simulink library "R9000_FirmwareLib"  (R2024b)
settings: Solver=VariableStepAuto  StartTime=0.0  StopTime=10.0  RelTol=1e-3
libraries: mbd_s32k3_io_library x82, mbd_s32k3_isr_library x15, mbd_s32k3_communication_library x10, mbd_s32k3_utility_library x1
(part 7 of 7)

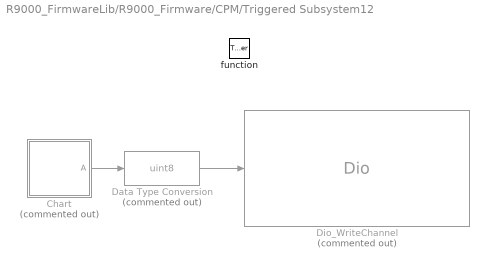
== system R9000_FirmwareLib/R9000_Firmware/CPM/Triggered Subsystem12 ==
  function : TriggerPort  TriggerType=function-call  VariantControl=Variant
  Chart : Stateflow chart  Commented=on  TreatAsAtomicUnit=on
  Data Type Conversion : DataTypeConversion  Commented=on  OutDataTypeStr=uint8  SaturateOnIntegerOverflow=off  RndMeth=Floor
  Dio_WriteChannel : mbd_s32k3_io_library/Dio  Commented=on  api_func=Dio_WriteChannel  channel=DI_DescentModeSwitch1  channel_group=No channel groups configured  port=PTA_H  gpioSim=on  text=<binary blob, 4879 chars, not shown>
      settings: SourceProductName=IO Blocks
  wires:
  Data Type Conversion/1 -> Dio_WriteChannel/1
  Chart/1(" A") -> Data Type Conversion/1

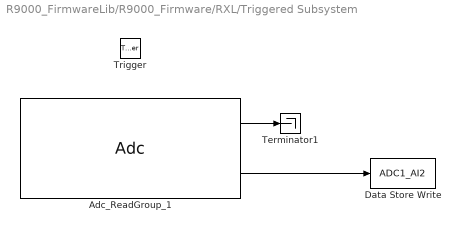
== system R9000_FirmwareLib/R9000_Firmware/RXL/Triggered Subsystem ==
  Trigger : TriggerPort  TriggerType=function-call  VariantControl=Variant
  Adc_ReadGroup_1 : mbd_s32k3_io_library/Adc  api_func=Adc_ReadGroup  hw_unit=AdcHwUnit_0  group=Adc0Group_0  channel=ADC_1_AI2  threshold_control=No ADC threshold control configured  power_state=ADC_FULL_POWER  ctu_unit=BctuHwUnit_0  ctu_trigger=AdcHwTrigger_0  ctu_fifo_idx=BctuResultFifos_0  text=<binary blob, 63510 chars, not shown>
      settings: SourceProductName=IO Blocks
  Data Store Write : DataStoreWrite  DataStoreName=ADC1_AI2  IndexOptions=Index vector (dialog)  Indices=1  OutputSizes=1
  Terminator1 : Terminator
  wires:
  Adc_ReadGroup_1/2 -> Data Store Write/1
  Adc_ReadGroup_1/1 -> Terminator1/1

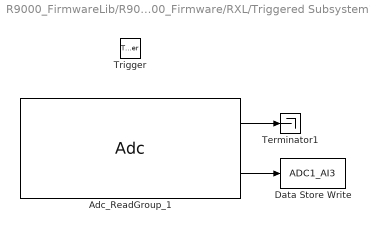
== system R9000_FirmwareLib/R9000_Firmware/RXL/Triggered Subsystem1 ==
  Trigger : TriggerPort  TriggerType=function-call  VariantControl=Variant
  Adc_ReadGroup_1 : mbd_s32k3_io_library/Adc  api_func=Adc_ReadGroup  hw_unit=AdcHwUnit_0  group=Adc0Group_1  channel=ADC_1_AI2  threshold_control=No ADC threshold control configured  power_state=ADC_FULL_POWER  ctu_unit=BctuHwUnit_0  ctu_trigger=AdcHwTrigger_0  ctu_fifo_idx=BctuResultFifos_0  text=<binary blob, 63510 chars, not shown>
      settings: SourceProductName=IO Blocks
  Data Store Write : DataStoreWrite  DataStoreName=ADC1_AI3  IndexOptions=Index vector (dialog)  Indices=1  OutputSizes=1
  Terminator1 : Terminator
  wires:
  Adc_ReadGroup_1/2 -> Data Store Write/1
  Adc_ReadGroup_1/1 -> Terminator1/1

== system R9000_FirmwareLib/R9000_Firmware/RXL/Triggered Subsystem10 ==
  Trigger : TriggerPort  TriggerType=function-call  VariantControl=Variant
  Adc_ReadGroup_1 : mbd_s32k3_io_library/Adc  api_func=Adc_ReadGroup  hw_unit=AdcHwUnit_0  group=Adc2Group_5  channel=ADC_1_AI2  threshold_control=No ADC threshold control configured  power_state=ADC_FULL_POWER  ctu_unit=BctuHwUnit_0  ctu_trigger=AdcHwTrigger_0  ctu_fifo_idx=BctuResultFifos_0  text=<binary blob, 63510 chars, not shown>
      settings: SourceProductName=IO Blocks
  Data Store Write : DataStoreWrite  DataStoreName=AdcSecondaryPressureSensor  IndexOptions=Index vector (dialog)  Indices=1  OutputSizes=1
  Terminator1 : Terminator
  wires:
  Adc_ReadGroup_1/2 -> Data Store Write/1
  Adc_ReadGroup_1/1 -> Terminator1/1

== system R9000_FirmwareLib/R9000_Firmware/RXL/Triggered Subsystem11 ==
  Trigger : TriggerPort  TriggerType=function-call  VariantControl=Variant
  Adc_ReadGroup_1 : mbd_s32k3_io_library/Adc  api_func=Adc_ReadGroup  hw_unit=AdcHwUnit_0  group=Adc2Group_6  channel=ADC_1_AI2  threshold_control=No ADC threshold control configured  power_state=ADC_FULL_POWER  ctu_unit=BctuHwUnit_0  ctu_trigger=AdcHwTrigger_0  ctu_fifo_idx=BctuResultFifos_0  text=<binary blob, 63510 chars, not shown>
      settings: SourceProductName=IO Blocks
  Data Store Write : DataStoreWrite  DataStoreName=AdcSumpOilTempSensor  IndexOptions=Index vector (dialog)  Indices=1  OutputSizes=1
  Terminator1 : Terminator
  wires:
  Adc_ReadGroup_1/2 -> Data Store Write/1
  Adc_ReadGroup_1/1 -> Terminator1/1

== system R9000_FirmwareLib/R9000_Firmware/RXL/Triggered Subsystem2 ==
  Trigger : TriggerPort  TriggerType=function-call  VariantControl=Variant
  Adc_ReadGroup_1 : mbd_s32k3_io_library/Adc  api_func=Adc_ReadGroup  hw_unit=AdcHwUnit_0  group=Adc0Group_2  channel=ADC_1_AI2  threshold_control=No ADC threshold control configured  power_state=ADC_FULL_POWER  ctu_unit=BctuHwUnit_0  ctu_trigger=AdcHwTrigger_0  ctu_fifo_idx=BctuResultFifos_0  text=<binary blob, 63510 chars, not shown>
      settings: SourceProductName=IO Blocks
  Data Store Write : DataStoreWrite  DataStoreName=ADC1_AI4  IndexOptions=Index vector (dialog)  Indices=1  OutputSizes=1
  Terminator1 : Terminator
  wires:
  Adc_ReadGroup_1/2 -> Data Store Write/1
  Adc_ReadGroup_1/1 -> Terminator1/1

== system R9000_FirmwareLib/R9000_Firmware/RXL/Triggered Subsystem3 ==
  Trigger : TriggerPort  TriggerType=function-call  VariantControl=Variant
  Adc_ReadGroup_1 : mbd_s32k3_io_library/Adc  api_func=Adc_ReadGroup  hw_unit=AdcHwUnit_0  group=Adc2Group_1  channel=ADC_1_AI2  threshold_control=No ADC threshold control configured  power_state=ADC_FULL_POWER  ctu_unit=BctuHwUnit_0  ctu_trigger=AdcHwTrigger_0  ctu_fifo_idx=BctuResultFifos_0  text=<binary blob, 63510 chars, not shown>
      settings: SourceProductName=IO Blocks
  Data Store Write : DataStoreWrite  DataStoreName=AdcGPSSensor  IndexOptions=Index vector (dialog)  Indices=1  OutputSizes=1
  Terminator1 : Terminator
  wires:
  Adc_ReadGroup_1/2 -> Data Store Write/1
  Adc_ReadGroup_1/1 -> Terminator1/1

== system R9000_FirmwareLib/R9000_Firmware/RXL/Triggered Subsystem4 ==
  Trigger : TriggerPort  TriggerType=function-call  VariantControl=Variant
  Adc_ReadGroup_1 : mbd_s32k3_io_library/Adc  api_func=Adc_ReadGroup  hw_unit=AdcHwUnit_0  group=Adc2Group_2  channel=ADC_1_AI2  threshold_control=No ADC threshold control configured  power_state=ADC_FULL_POWER  ctu_unit=BctuHwUnit_0  ctu_trigger=AdcHwTrigger_0  ctu_fifo_idx=BctuResultFifos_0  text=<binary blob, 63510 chars, not shown>
      settings: SourceProductName=IO Blocks
  Data Store Write : DataStoreWrite  DataStoreName=AdcLCPressureSensor  IndexOptions=Index vector (dialog)  Indices=1  OutputSizes=1
  Terminator1 : Terminator
  wires:
  Adc_ReadGroup_1/2 -> Data Store Write/1
  Adc_ReadGroup_1/1 -> Terminator1/1

== system R9000_FirmwareLib/R9000_Firmware/RXL/Triggered Subsystem5 ==
  Trigger : TriggerPort  TriggerType=function-call  VariantControl=Variant
  Adc_ReadGroup_1 : mbd_s32k3_io_library/Adc  api_func=Adc_ReadGroup  hw_unit=AdcHwUnit_0  group=Adc2Group_0  channel=ADC_1_AI2  threshold_control=No ADC threshold control configured  power_state=ADC_FULL_POWER  ctu_unit=BctuHwUnit_0  ctu_trigger=AdcHwTrigger_0  ctu_fifo_idx=BctuResultFifos_0  text=<binary blob, 63510 chars, not shown>
      settings: SourceProductName=IO Blocks
  Data Store Write : DataStoreWrite  DataStoreName=AdcPilotPressureSensor  IndexOptions=Index vector (dialog)  Indices=1  OutputSizes=1
  Terminator1 : Terminator
  wires:
  Adc_ReadGroup_1/2 -> Data Store Write/1
  Adc_ReadGroup_1/1 -> Terminator1/1

== system R9000_FirmwareLib/R9000_Firmware/RXL/Triggered Subsystem6 ==
  Trigger : TriggerPort  TriggerType=function-call  VariantControl=Variant
  Adc_ReadGroup_1 : mbd_s32k3_io_library/Adc  api_func=Adc_ReadGroup  hw_unit=AdcHwUnit_0  group=Adc0Group_3  channel=ADC_1_AI2  threshold_control=No ADC threshold control configured  power_state=ADC_FULL_POWER  ctu_unit=BctuHwUnit_0  ctu_trigger=AdcHwTrigger_0  ctu_fifo_idx=BctuResultFifos_0  text=<binary blob, 63510 chars, not shown>
      settings: SourceProductName=IO Blocks
  Data Store Write : DataStoreWrite  DataStoreName=AdcATMPressureSensor  IndexOptions=Index vector (dialog)  Indices=1  OutputSizes=1
  Terminator1 : Terminator
  wires:
  Adc_ReadGroup_1/2 -> Data Store Write/1
  Adc_ReadGroup_1/1 -> Terminator1/1

== system R9000_FirmwareLib/R9000_Firmware/RXL/Triggered Subsystem7 ==
  Trigger : TriggerPort  TriggerType=function-call  VariantControl=Variant
  Adc_ReadGroup_1 : mbd_s32k3_io_library/Adc  api_func=Adc_ReadGroup  hw_unit=AdcHwUnit_0  group=Adc0Group_4  channel=ADC_1_AI2  threshold_control=No ADC threshold control configured  power_state=ADC_FULL_POWER  ctu_unit=BctuHwUnit_0  ctu_trigger=AdcHwTrigger_0  ctu_fifo_idx=BctuResultFifos_0  text=<binary blob, 63510 chars, not shown>
      settings: SourceProductName=IO Blocks
  Data Store Write : DataStoreWrite  DataStoreName=ADC1_AI1  IndexOptions=Index vector (dialog)  Indices=1  OutputSizes=1
  Terminator1 : Terminator
  wires:
  Adc_ReadGroup_1/2 -> Data Store Write/1
  Adc_ReadGroup_1/1 -> Terminator1/1

== system R9000_FirmwareLib/R9000_Firmware/RXL/Triggered Subsystem8 ==
  Trigger : TriggerPort  TriggerType=function-call  VariantControl=Variant
  Adc_ReadGroup_1 : mbd_s32k3_io_library/Adc  api_func=Adc_ReadGroup  hw_unit=AdcHwUnit_0  group=Adc2Group_3  channel=ADC_1_AI2  threshold_control=No ADC threshold control configured  power_state=ADC_FULL_POWER  ctu_unit=BctuHwUnit_0  ctu_trigger=AdcHwTrigger_0  ctu_fifo_idx=BctuResultFifos_0  text=<binary blob, 63510 chars, not shown>
      settings: SourceProductName=IO Blocks
  Data Store Write : DataStoreWrite  DataStoreName=AdcLinePressureSensor  IndexOptions=Index vector (dialog)  Indices=1  OutputSizes=1
  Terminator1 : Terminator
  wires:
  Adc_ReadGroup_1/2 -> Data Store Write/1
  Adc_ReadGroup_1/1 -> Terminator1/1

== system R9000_FirmwareLib/R9000_Firmware/RXL/Triggered Subsystem9 ==
  Trigger : TriggerPort  TriggerType=function-call  VariantControl=Variant
  Adc_ReadGroup_1 : mbd_s32k3_io_library/Adc  api_func=Adc_ReadGroup  hw_unit=AdcHwUnit_0  group=Adc2Group_4  channel=ADC_1_AI2  threshold_control=No ADC threshold control configured  power_state=ADC_FULL_POWER  ctu_unit=BctuHwUnit_0  ctu_trigger=AdcHwTrigger_0  ctu_fifo_idx=BctuResultFifos_0  text=<binary blob, 63510 chars, not shown>
      settings: SourceProductName=IO Blocks
  Data Store Write : DataStoreWrite  DataStoreName=AdcPrimaryPressureSensor  IndexOptions=Index vector (dialog)  Indices=1  OutputSizes=1
  Terminator1 : Terminator
  wires:
  Adc_ReadGroup_1/2 -> Data Store Write/1
  Adc_ReadGroup_1/1 -> Terminator1/1

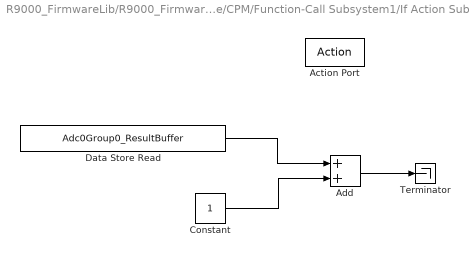
== system R9000_FirmwareLib/R9000_Firmware/CPM/Function-Call Subsystem1/If Action Subsystem ==
  Action Port : ActionPort
  Add : Sum
  Constant : Constant  OutDataTypeStr=uint8
  Data Store Read : DataStoreRead  DataStoreName=Adc0Group0_ResultBuffer  IndexOptions=Index vector (dialog)  Indices=1  OutputSizes=1
  Terminator : Terminator
  wires:
  Data Store Read/1 -> Add/1
  Constant/1 -> Add/2
  Add/1 -> Terminator/1

== system R9000_FirmwareLib/R9000_Firmware/CPM/Function-Call Subsystem3/If Action Subsystem ==
  Action Port : ActionPort
  Chart : Stateflow chart  TreatAsAtomicUnit=on
  Data Type Conversion : DataTypeConversion  OutDataTypeStr=uint8  SaturateOnIntegerOverflow=off  RndMeth=Floor
  Terminator : Terminator
  wires:
  Chart/1(" A") -> Data Type Conversion/1
  Data Type Conversion/1 -> Terminator/1

== stateflow chart R9000_FirmwareLib/R9000_Firmware/CPM/Function-Call Subsystem3/If Action Subsystem/Chart ==
  state A
  state A1
  (default) -> A
  A -> A1
  A1 -> A
  data: A output boolean [0..1]

== stateflow chart R9000_FirmwareLib/R9000_Firmware/CPM/Triggered Subsystem12/Chart ==
  state A
  state A1
  (default) -> A
  A -> A1
  A1 -> A
  data: A output boolean [0..1]
